simulink model "Simulatore_COMAU_UBHAND_IV_manipolazione_interna"  (Simulink 7.6) (continued)
== system Simulatore_COMAU_UBHAND_IV_manipolazione_interna/Work Space Control/Grasp Planner/box/presa dal basso == (continued)
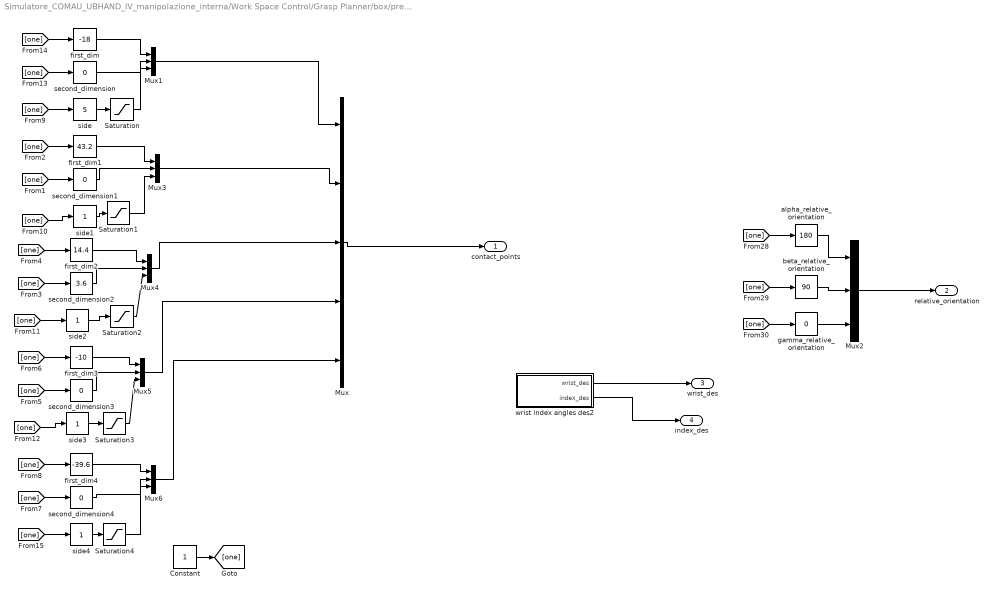
== system Simulatore_COMAU_UBHAND_IV_manipolazione_interna/Work Space Control/Grasp Planner/box/presa dall'alto ==
  Constant : Constant
  Mux : Mux  Inputs=5
  Mux{1..6} (6 alike) : Mux  Inputs=3
  Saturation{,1..4} (5 alike) : Saturate  UpperLimit=6  LowerLimit=1
  alpha_relative_orientation : simulink/Math Operations/Slider Gain  low=-180  gain=180  high=180
  beta_relative_orientation : simulink/Math Operations/Slider Gain  low=-180  gain=90  high=180
  first_dim : simulink/Math Operations/Slider Gain  low=-180  gain=-18  high=180
  first_dim1 : simulink/Math Operations/Slider Gain  low=-180  gain=43.2  high=180
  first_dim2 : simulink/Math Operations/Slider Gain  low=-180  gain=14.4  high=180
  first_dim3 : simulink/Math Operations/Slider Gain  low=-100  gain=-10  high=100
  first_dim4 : simulink/Math Operations/Slider Gain  low=-180  gain=-39.6  high=180
  gamma_relative_orientation : simulink/Math Operations/Slider Gain  low=-180  gain=0  high=180
  second_dimension{,1,3..4} (4 alike) : simulink/Math Operations/Slider Gain  low=-180  gain=0  high=180
  second_dimension2 : simulink/Math Operations/Slider Gain  low=-180  gain=3.6  high=180
  side : simulink/Math Operations/Slider Gain  low=-94  gain=5  high=6
  side{1..4} (4 alike) : simulink/Math Operations/Slider Gain  low=-94  gain=1  high=6
  wrist index angles des2 : Subsystem
  contact_points : Outport
  relative_orientation : Outport  Port=2
  wrist_des : Outport  Port=3
  index_des : Outport  Port=4
  wires:
  [one] -> alpha_relative_orientation/1
  [one] -> beta_relative_orientation/1
  [one] -> gamma_relative_orientation/1
  alpha_relative_orientation/1 -> Mux2/1
  beta_relative_orientation/1 -> Mux2/2
  gamma_relative_orientation/1 -> Mux2/3
  Mux2/1 -> relative_orientation/1
  Constant/1 -> [one]
  wrist index angles des2/1(wrist_des) -> wrist_des/1
  wrist index angles des2/2(index_des) -> index_des/1
  Mux/1 -> contact_points/1
  [one] -> second_dimension/1
  [one] -> first_dim/1
  first_dim/1 -> Mux1/1
  second_dimension/1 -> Mux1/2
  Mux1/1 -> Mux/1
  side/1 -> Saturation/1
  [one] -> second_dimension1/1
  [one] -> first_dim1/1
  first_dim1/1 -> Mux3/1
  second_dimension1/1 -> Mux3/2
  side1/1 -> Saturation1/1
  [one] -> second_dimension2/1
  [one] -> first_dim2/1
  first_dim2/1 -> Mux4/1
  second_dimension2/1 -> Mux4/2
  side2/1 -> Saturation2/1
  [one] -> second_dimension3/1
  [one] -> first_dim3/1
  first_dim3/1 -> Mux5/1
  second_dimension3/1 -> Mux5/2
  side3/1 -> Saturation3/1
  [one] -> second_dimension4/1
  [one] -> first_dim4/1
  first_dim4/1 -> Mux6/1
  second_dimension4/1 -> Mux6/2
  side4/1 -> Saturation4/1
  Mux3/1 -> Mux/2
  Mux4/1 -> Mux/3
  Mux5/1 -> Mux/4
  Mux6/1 -> Mux/5
  [one] -> side/1
  [one] -> side1/1
  [one] -> side2/1
  [one] -> side3/1
  [one] -> side4/1
  Saturation/1 -> Mux1/3
  Saturation1/1 -> Mux3/3
  Saturation2/1 -> Mux4/3
  Saturation3/1 -> Mux5/3
  Saturation4/1 -> Mux6/3

== system Simulatore_COMAU_UBHAND_IV_manipolazione_interna/Work Space Control/Grasp Planner/box/punti di contatto box ==
  parallelipedo_size : Inport
  sfera_pos : Inport  Port=2
  object_hand_relative_orientation : Inport  Port=3
  object_motion_parameters : Inport  Port=4
  teta_phi : Inport  Port=5
  Constant : Constant
  Demux : Demux  Outputs=5
  object rotation matrix : Subsystem
  punti di contatto sfera : Subsystem
  contact_tips_xyz : Outport
  wires:
  parallelipedo_size/1 -> punti di contatto sfera/6(box_size)
  punti di contatto sfera/1(contact_tips_xyz) -> contact_tips_xyz/1
  Constant/1 -> [one]
  object rotation matrix/1(T_object_center) -> punti di contatto sfera/7(T_object)
  sfera_pos/1 -> object rotation matrix/1(box_pos)
  object_hand_relative_orientation/1 -> object rotation matrix/2(object_hand_relative_orientation)
  object_motion_parameters/1 -> object rotation matrix/3(object_motion_parameters)
  teta_phi/1 -> Demux/1
  Demux/1 -> punti di contatto sfera/1(teta/z thumb)
  Demux/2 -> punti di contatto sfera/2(teta/z index)
  Demux/3 -> punti di contatto sfera/3(teta/z middle)
  Demux/4 -> punti di contatto sfera/4(teta/z ring)
  Demux/5 -> punti di contatto sfera/5(teta/z little)

== system Simulatore_COMAU_UBHAND_IV_manipolazione_interna/Work Space Control/Grasp Planner/cilindro/Subsystem ==
  obj_rotat_manip : Inport
  obj_trasl_manip : Inport  Port=2
  Constant1 : Constant  Value=[0 0 0 1]  VectorParams1D=off
  Matrix Concatenate : Concatenate  Mode=Multidimensional array  ConcatenateDimension=2
  Matrix Concatenate1 : Concatenate  Mode=Multidimensional array
  R_end_effector : Subsystem
  obj_manip : Outport
  tag scope: R_cilindro_manip=global
  wires:
  Constant1/1 -> Matrix Concatenate1/2
  Matrix Concatenate/1 -> Matrix Concatenate1/1
  R_end_effector/1(R_end_effector) -> Matrix Concatenate/1, [R_cilindro_manip]
  obj_rotat_manip/1 -> R_end_effector/1(alpha,beta,gamma)
  obj_trasl_manip/1 -> Matrix Concatenate/2
  Matrix Concatenate1/1 -> obj_manip/1

== system Simulatore_COMAU_UBHAND_IV_manipolazione_interna/Work Space Control/Grasp Planner/cilindro/presa da destra ==
  Add{,1..3,6..8} (7 alike) : Sum
  Constant : Constant
  Mux : Mux  Inputs=10
  Mux2 : Mux  Inputs=3
  alpha_relative_orientation : simulink/Math Operations/Slider Gain  low=-180  gain=90  high=180
  beta_relative_orientation : simulink/Math Operations/Slider Gain  low=-180  gain=90  high=180
  gamma_relative_orientation : simulink/Math Operations/Slider Gain  low=-180  gain=90  high=180
  teta : simulink/Math Operations/Slider Gain  low=-180  gain=115.2  high=180
  teta1 : simulink/Math Operations/Slider Gain  low=-180  gain=32.4  high=180
  teta2 : simulink/Math Operations/Slider Gain  low=-180  gain=0  high=180
  teta3 : simulink/Math Operations/Slider Gain  low=-180  gain=0  high=180
  teta4 : simulink/Math Operations/Slider Gain  low=-180  gain=0  high=180
  wrist index angles des2 : Subsystem
  z : simulink/Math Operations/Slider Gain  low=-100  gain=60  high=500
  z1 : simulink/Math Operations/Slider Gain  low=-100  gain=-4e-006  high=500
  z2 : simulink/Math Operations/Slider Gain  low=-100  gain=-24  high=500
  z3 : simulink/Math Operations/Slider Gain  low=-100  gain=-48  high=500
  z4 : simulink/Math Operations/Slider Gain  low=-100  gain=-72  high=500
  contact_points : Outport
  relative_orientation : Outport  Port=2
  wrist_des : Outport  Port=3
  index_des : Outport  Port=4
  wires:
  Mux2/1 -> relative_orientation/1
  gamma_relative_orientation/1 -> Mux2/3
  beta_relative_orientation/1 -> Mux2/2
  alpha_relative_orientation/1 -> Mux2/1
  [one] -> gamma_relative_orientation/1
  [one] -> beta_relative_orientation/1
  [one] -> alpha_relative_orientation/1
  Mux/1 -> contact_points/1
  [one] -> teta4/1
  [one] -> z4/1
  [one] -> teta3/1
  [one] -> z3/1
  [one] -> teta2/1
  [one] -> z2/1
  [one] -> teta1/1
  [one] -> z1/1
  [one] -> teta/1
  [one] -> z/1
  Constant/1 -> [one]
  wrist index angles des2/1(wrist_des) -> wrist_des/1
  wrist index angles des2/2(index_des) -> index_des/1
  z4/1 -> Add3/2
  Add3/1 -> Mux/10
  z3/1 -> Add2/2
  Add2/1 -> Mux/8
  z2/1 -> Add1/2
  Add1/1 -> Mux/6
  z1/1 -> Mux/4, Add/2, Add1/1, Add2/1, Add3/1
  teta1/1 -> Mux/3, Add6/1, Add7/1, Add8/1
  teta4/1 -> Add8/2
  Add8/1 -> Mux/9
  Add7/1 -> Mux/7
  teta3/1 -> Add7/2
  teta2/1 -> Add6/2
  Add6/1 -> Mux/5
  teta/1 -> Mux/1
  z/1 -> Add/1
  Add/1 -> Mux/2

== system Simulatore_COMAU_UBHAND_IV_manipolazione_interna/Work Space Control/Grasp Planner/cilindro/presa dal basso ==
  Add{,1..4,6..8} (8 alike) : Sum
  Constant : Constant
  Mux : Mux  Inputs=10
  Mux2 : Mux  Inputs=3
  alpha_relative_orientation : simulink/Math Operations/Slider Gain  low=-180  gain=180  high=180
  beta_relative_orientation : simulink/Math Operations/Slider Gain  low=-180  gain=90  high=180
  gamma_relative_orientation : simulink/Math Operations/Slider Gain  low=-180  gain=90  high=180
  teta : simulink/Math Operations/Slider Gain  low=-180  gain=-46.8  high=180
  teta1 : simulink/Math Operations/Slider Gain  low=-180  gain=-46.8  high=180
  teta2 : simulink/Math Operations/Slider Gain  low=-180  gain=39.6  high=180
  teta3 : simulink/Math Operations/Slider Gain  low=-180  gain=79.2  high=180
  teta4 : simulink/Math Operations/Slider Gain  low=-180  gain=126  high=180
  wrist index angles des2 : Subsystem
  z : simulink/Math Operations/Slider Gain  low=-100  gain=-42  high=500
  z1 : simulink/Math Operations/Slider Gain  low=-100  gain=-18  high=500
  z2 : simulink/Math Operations/Slider Gain  low=-100  gain=-6  high=500
  z3 : simulink/Math Operations/Slider Gain  low=-100  gain=-6  high=500
  z4 : simulink/Math Operations/Slider Gain  low=-100  gain=-12  high=500
  contact_points : Outport
  relative_orientation : Outport  Port=2
  wrist_des : Outport  Port=3
  index_des : Outport  Port=4
  wires:
  [one] -> z/1
  [one] -> teta/1
  [one] -> z1/1
  [one] -> teta1/1
  [one] -> z2/1
  [one] -> teta2/1
  [one] -> z3/1
  [one] -> teta3/1
  [one] -> z4/1
  [one] -> teta4/1
  [one] -> alpha_relative_orientation/1
  [one] -> beta_relative_orientation/1
  [one] -> gamma_relative_orientation/1
  alpha_relative_orientation/1 -> Mux2/1
  beta_relative_orientation/1 -> Mux2/2
  gamma_relative_orientation/1 -> Mux2/3
  Mux2/1 -> relative_orientation/1
  Constant/1 -> [one]
  wrist index angles des2/1(wrist_des) -> wrist_des/1
  wrist index angles des2/2(index_des) -> index_des/1
  Mux/1 -> contact_points/1
  z4/1 -> Add3/2
  Add3/1 -> Mux/10
  z3/1 -> Add2/2
  Add2/1 -> Mux/8
  z2/1 -> Add1/2
  Add1/1 -> Mux/6
  z1/1 -> Mux/4, Add/2, Add1/1, Add2/1, Add3/1
  teta1/1 -> Mux/3, Add6/1, Add7/1, Add8/1, Add4/2
  teta4/1 -> Add8/2
  Add8/1 -> Mux/9
  Add7/1 -> Mux/7
  teta3/1 -> Add7/2
  teta2/1 -> Add6/2
  Add6/1 -> Mux/5
  z/1 -> Add/1
  Add/1 -> Mux/2
  teta/1 -> Add4/1
  Add4/1 -> Mux/1

== system Simulatore_COMAU_UBHAND_IV_manipolazione_interna/Work Space Control/Grasp Planner/cilindro/presa dall'alto ==
  Add{,1..4,6..8} (8 alike) : Sum
  Constant : Constant
  Mux : Mux  Inputs=10
  Mux2 : Mux  Inputs=3
  alpha_relative_orientation : simulink/Math Operations/Slider Gain  low=-180  gain=180  high=180
  beta_relative_orientation : simulink/Math Operations/Slider Gain  low=-180  gain=90  high=180
  gamma_relative_orientation : simulink/Math Operations/Slider Gain  low=-180  gain=0  high=180
  index_angles_des : Display
  teta : simulink/Math Operations/Slider Gain  low=-180  gain=86.4  high=180
  teta1 : simulink/Math Operations/Slider Gain  low=-180  gain=54  high=180
  teta2 : simulink/Math Operations/Slider Gain  low=-180  gain=-36  high=180
  teta3 : simulink/Math Operations/Slider Gain  low=-180  gain=-79.2  high=180
  teta4 : simulink/Math Operations/Slider Gain  low=-180  gain=-147.6  high=180
  wrist index angles des2 : Subsystem
  wrist_angles_des : Display
  z : simulink/Math Operations/Slider Gain  low=-100  gain=-4  high=100
  z1 : simulink/Math Operations/Slider Gain  low=-100  gain=180  high=500
  z2 : simulink/Math Operations/Slider Gain  low=-100  gain=0  high=100
  z3 : simulink/Math Operations/Slider Gain  low=-100  gain=0  high=500
  z4 : simulink/Math Operations/Slider Gain  low=-200  gain=0  high=500
  contact_points : Outport
  relative_orientation : Outport  Port=2
  wrist_des : Outport  Port=3
  index_des : Outport  Port=4
  wires:
  [one] -> z/1
  [one] -> teta/1
  [one] -> z1/1
  [one] -> teta1/1
  [one] -> z2/1
  [one] -> teta2/1
  [one] -> z3/1
  [one] -> teta3/1
  [one] -> z4/1
  [one] -> teta4/1
  [one] -> alpha_relative_orientation/1
  [one] -> beta_relative_orientation/1
  [one] -> gamma_relative_orientation/1
  alpha_relative_orientation/1 -> Mux2/1
  beta_relative_orientation/1 -> Mux2/2
  gamma_relative_orientation/1 -> Mux2/3
  Mux2/1 -> relative_orientation/1
  Constant/1 -> [one]
  wrist index angles des2/1(wrist_des) -> wrist_des/1, wrist_angles_des/1
  wrist index angles des2/2(index_des) -> index_des/1, index_angles_des/1
  Mux/1 -> contact_points/1
  z4/1 -> Add3/2
  Add3/1 -> Mux/10
  z3/1 -> Add2/2
  Add2/1 -> Mux/8
  z2/1 -> Add1/2
  Add1/1 -> Mux/6
  z1/1 -> Mux/4, Add/2, Add1/1, Add2/1, Add3/1
  teta1/1 -> Mux/3, Add6/1, Add7/1, Add8/1, Add4/2
  teta4/1 -> Add8/2
  Add8/1 -> Mux/9
  Add7/1 -> Mux/7
  teta3/1 -> Add7/2
  teta2/1 -> Add6/2
  Add6/1 -> Mux/5
  z/1 -> Add/1
  Add/1 -> Mux/2
  teta/1 -> Add4/1
  Add4/1 -> Mux/1

== system Simulatore_COMAU_UBHAND_IV_manipolazione_interna/Work Space Control/Grasp Planner/cilindro/punti di contatto cilindro ==
  cilindro_size : Inport
  cilindro_pos : Inport  Port=2
  teta_z : Inport  Port=3
  object_hand_relative_orientation : Inport  Port=4
  object_motion_parameters : Inport  Port=5
  offset : Inport  Port=6
  object_manipulation : Inport  Port=7
  Angle Conversion{,1..4} (5 alike) : aerolibconvert2/Angle Conversion  IU=deg  OU=rad
  Constant : Constant
  Demux : Demux  Outputs=10
  Mux{,1..4} (5 alike) : Mux  Inputs=2
  object rotation matrix : Subsystem
  punti di contatto cilindro : Subsystem
  contact_tips_xyz : Outport
  index_xyz_0 : Outport  Port=2
  wires:
  cilindro_size/1 -> punti di contatto cilindro/6(cilindro_size)
  Mux/1 -> punti di contatto cilindro/1(teta/z thumb)
  Mux1/1 -> punti di contatto cilindro/2(teta/z index)
  Mux2/1 -> punti di contatto cilindro/3(teta/z middle)
  Mux3/1 -> punti di contatto cilindro/4(teta/z ring)
  Mux4/1 -> punti di contatto cilindro/5(teta/z little)
  punti di contatto cilindro/1(contact_tips_xyz) -> contact_tips_xyz/1
  Constant/1 -> [one]
  Angle Conversion/1 -> Mux/1
  Angle Conversion1/1 -> Mux1/1
  Angle Conversion2/1 -> Mux2/1
  Angle Conversion3/1 -> Mux3/1
  Angle Conversion4/1 -> Mux4/1
  object rotation matrix/1(T_object_center) -> punti di contatto cilindro/7(T_object)
  teta_z/1 -> Demux/1
  object_hand_relative_orientation/1 -> object rotation matrix/2(object_hand_relative_orientation)
  object_motion_parameters/1 -> object rotation matrix/3(object_motion_parameters)
  Demux/1 -> Angle Conversion/1
  Demux/2 -> Mux/2
  Demux/3 -> Angle Conversion1/1
  Demux/4 -> Mux1/2
  Demux/5 -> Angle Conversion2/1
  Demux/6 -> Mux2/2
  Demux/7 -> Angle Conversion3/1
  Demux/8 -> Mux3/2
  Demux/9 -> Angle Conversion4/1
  Demux/10 -> Mux4/2
  offset/1 -> punti di contatto cilindro/8(offset)
  cilindro_pos/1 -> object rotation matrix/1(cilindro_pos)
  object_manipulation/1 -> punti di contatto cilindro/9(object_manipulation)
  punti di contatto cilindro/2(index_xyz_0) -> index_xyz_0/1

== system Simulatore_COMAU_UBHAND_IV_manipolazione_interna/Work Space Control/Grasp Planner/sfera/presa da destra ==
  Constant : Constant
  Mux2 : Mux  Inputs=3
  alpha_relative_orientation : simulink/Math Operations/Slider Gain  low=-180  gain=180  high=180
  beta_relative_orientation : simulink/Math Operations/Slider Gain  low=-180  gain=90  high=180
  gamma_relative_orientation : simulink/Math Operations/Slider Gain  low=-180  gain=90  high=180
  relative_orientation : Outport
  wires:
  Constant/1 -> [one]
  [one] -> alpha_relative_orientation/1
  [one] -> beta_relative_orientation/1
  [one] -> gamma_relative_orientation/1
  alpha_relative_orientation/1 -> Mux2/1
  beta_relative_orientation/1 -> Mux2/2
  gamma_relative_orientation/1 -> Mux2/3
  Mux2/1 -> relative_orientation/1

== system Simulatore_COMAU_UBHAND_IV_manipolazione_interna/Work Space Control/Grasp Planner/sfera/presa dal basso ==
  Constant : Constant
  Mux2 : Mux  Inputs=3
  alpha_relative_orientation : simulink/Math Operations/Slider Gain  low=-180  gain=-90  high=180
  beta_relative_orientation : simulink/Math Operations/Slider Gain  low=-180  gain=90  high=180
  gamma_relative_orientation : simulink/Math Operations/Slider Gain  low=-180  gain=90  high=180
  relative_orientation : Outport
  wires:
  Constant/1 -> [one]
  Mux2/1 -> relative_orientation/1
  gamma_relative_orientation/1 -> Mux2/3
  beta_relative_orientation/1 -> Mux2/2
  alpha_relative_orientation/1 -> Mux2/1
  [one] -> gamma_relative_orientation/1
  [one] -> beta_relative_orientation/1
  [one] -> alpha_relative_orientation/1

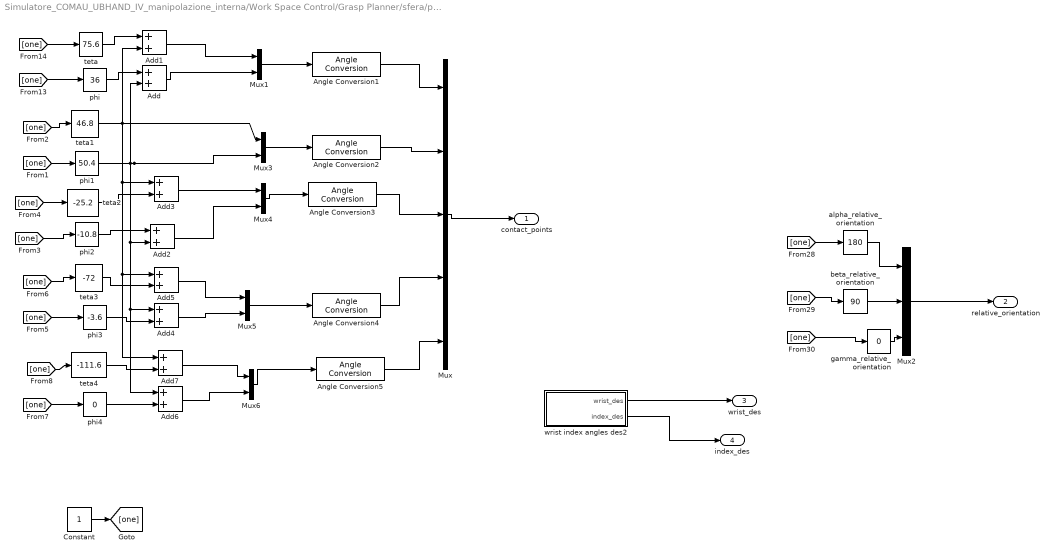
== system Simulatore_COMAU_UBHAND_IV_manipolazione_interna/Work Space Control/Grasp Planner/sfera/presa dall'alto ==
  Add{,1..7} (8 alike) : Sum
  Angle Conversion{1..5} (5 alike) : aerolibconvert2/Angle Conversion  IU=deg  OU=rad
  Constant : Constant
  Mux : Mux  Inputs=5
  Mux{1,3..6} (5 alike) : Mux  Inputs=2
  Mux2 : Mux  Inputs=3
  alpha_relative_orientation : simulink/Math Operations/Slider Gain  low=-180  gain=180  high=180
  beta_relative_orientation : simulink/Math Operations/Slider Gain  low=-180  gain=90  high=180
  gamma_relative_orientation : simulink/Math Operations/Slider Gain  low=-180  gain=0  high=180
  phi : simulink/Math Operations/Slider Gain  low=-180  gain=36  high=180
  phi1 : simulink/Math Operations/Slider Gain  low=-180  gain=50.4  high=180
  phi2 : simulink/Math Operations/Slider Gain  low=-180  gain=-10.8  high=180
  phi3 : simulink/Math Operations/Slider Gain  low=-180  gain=-3.6  high=180
  phi4 : simulink/Math Operations/Slider Gain  low=-180  gain=0  high=180
  teta : simulink/Math Operations/Slider Gain  low=-180  gain=75.6  high=180
  teta1 : simulink/Math Operations/Slider Gain  low=-180  gain=46.8  high=180
  teta2 : simulink/Math Operations/Slider Gain  low=-180  gain=-25.2  high=180
  teta3 : simulink/Math Operations/Slider Gain  low=-180  gain=-72  high=180
  teta4 : simulink/Math Operations/Slider Gain  low=-180  gain=-111.6  high=180
  wrist index angles des2 : Subsystem
  contact_points : Outport
  relative_orientation : Outport  Port=2
  wrist_des : Outport  Port=3
  index_des : Outport  Port=4
  wires:
  Mux/1 -> contact_points/1
  wrist index angles des2/2(index_des) -> index_des/1
  wrist index angles des2/1(wrist_des) -> wrist_des/1
  Constant/1 -> [one]
  Mux2/1 -> relative_orientation/1
  gamma_relative_orientation/1 -> Mux2/3
  beta_relative_orientation/1 -> Mux2/2
  alpha_relative_orientation/1 -> Mux2/1
  [one] -> gamma_relative_orientation/1
  [one] -> beta_relative_orientation/1
  [one] -> alpha_relative_orientation/1
  [one] -> phi/1
  [one] -> teta/1
  Mux1/1 -> Angle Conversion1/1
  [one] -> phi1/1
  [one] -> teta1/1
  teta1/1 -> Mux3/1, Add1/2, Add3/1, Add5/1, Add7/1
  [one] -> phi2/1
  [one] -> teta2/1
  [one] -> phi3/1
  [one] -> teta3/1
  [one] -> phi4/1
  [one] -> teta4/1
  Mux3/1 -> Angle Conversion2/1
  Mux4/1 -> Angle Conversion3/1
  Mux5/1 -> Angle Conversion4/1
  Mux6/1 -> Angle Conversion5/1
  phi1/1 -> Mux3/2, Add/2, Add6/1, Add4/1, Add2/2
  Angle Conversion1/1 -> Mux/1
  Angle Conversion2/1 -> Mux/2
  Angle Conversion3/1 -> Mux/3
  Angle Conversion4/1 -> Mux/4
  Angle Conversion5/1 -> Mux/5
  Add/1 -> Mux1/2
  Add1/1 -> Mux1/1
  teta4/1 -> Add7/2
  phi4/1 -> Add6/2
  Add6/1 -> Mux6/2
  Add7/1 -> Mux6/1
  Add4/1 -> Mux5/2
  Add5/1 -> Mux5/1
  Add2/1 -> Mux4/2
  Add3/1 -> Mux4/1
  phi/1 -> Add/1
  teta/1 -> Add1/1
  teta2/1 -> Add3/2
  phi3/1 -> Add4/2
  teta3/1 -> Add5/2
  phi2/1 -> Add2/1

== system Simulatore_COMAU_UBHAND_IV_manipolazione_interna/Work Space Control/Grasp Planner/sfera/punti di contatto sfera ==
  sfera_size : Inport
  sfera_pos : Inport  Port=2
  object_hand_relative_orientation : Inport  Port=3
  object_motion_parameters : Inport  Port=4
  teta_phi : Inport  Port=5
  Constant : Constant
  Demux : Demux  Outputs=5
  object rotation matrix : Subsystem
  punti di contatto sfera : Subsystem
  contact_tips_xyz : Outport
  wires:
  sfera_size/1 -> punti di contatto sfera/6(sfera_size)
  Demux/1 -> punti di contatto sfera/1(teta/z thumb)
  Demux/2 -> punti di contatto sfera/2(teta/z index)
  Demux/3 -> punti di contatto sfera/3(teta/z middle)
  Demux/5 -> punti di contatto sfera/5(teta/z little)
  Constant/1 -> [one]
  punti di contatto sfera/1(contact_tips_xyz) -> contact_tips_xyz/1
  object_motion_parameters/1 -> object rotation matrix/3(object_motion_parameters)
  object_hand_relative_orientation/1 -> object rotation matrix/2(object_hand_relative_orientation)
  sfera_pos/1 -> object rotation matrix/1(sfera_pos)
  object rotation matrix/1(T_object_center) -> punti di contatto sfera/7(T_object)
  teta_phi/1 -> Demux/1
  Demux/4 -> punti di contatto sfera/4(teta/z ring)

== system Simulatore_COMAU_UBHAND_IV_manipolazione_interna/Work Space Control/Inverse_kinematic/Inverse_kinematic_comau/R_0index ==
  wrist_angles_des1 : Inport
  index_angles_des1 : Inport  Port=2
  T_0_index : Inport  Port=3
  T_0_object : Inport  Port=4
  Matrix Multiply{1..4} (4 alike) : Product  Multiplication=Matrix(*)
  Multiport Switch : MultiPortSwitch  Inputs=2  zeroidx=off
  Multiport Switch1 : MultiPortSwitch  Inputs=2  zeroidx=off
  Multiport Switch2 : MultiPortSwitch  Inputs=2  zeroidx=off
  T6H : Constant  Value=T6HAND  VectorParams1D=off
  T6H1 : Constant  Value=T6HAND  VectorParams1D=off
  T_0_object1 : InportShadow  Port=4
  T_0index : Subsystem
  T_6_index_^-1 : Subsystem
  T_6_object^-1 : Subsystem
  T_index_object : Subsystem
  T_06 : Outport
  T_0H : Outport  Port=2
  tag scope: T_0EE=global
  wires:
  index_angles_des1/1 -> T_6_index_^-1/2(index_angles_des), T_0index/2(index_angles_des), T_6_object^-1/2(index_angles_des)
  Matrix Multiply1/1 -> Matrix Multiply2/1, Multiport Switch/2
  T6H/1 -> Matrix Multiply2/2
  wrist_angles_des1/1 -> T_0index/1(wrist_angles_des), T_6_index_^-1/1(wrist_angles_des), T_6_object^-1/1(wrist_angles_des)
  T_0_index/1 -> T_0index/3(T_0_end_effector), Multiport Switch2/2
  T_0index/1(T_0index) -> Matrix Multiply1/1, T_index_object/1(T_0_index)
  T_6_index_^-1/1(T_6_index^-1) -> Matrix Multiply1/2
  T_0_object/1 -> T_index_object/2(T_0_object), Multiport Switch2/3
  T_index_object/1(T_index_object) -> T_6_object^-1/3(T_index_object)
  T_0_object1/1 -> Matrix Multiply3/1
  T_6_object^-1/1(T_6_object^-1) -> Matrix Multiply3/2
  Multiport Switch/1 -> T_06/1
  Matrix Multiply3/1 -> Multiport Switch/3, Matrix Multiply4/1
  T6H1/1 -> Matrix Multiply4/2
  Matrix Multiply4/1 -> Multiport Switch1/3
  Matrix Multiply2/1 -> Multiport Switch1/2
  Multiport Switch1/1 -> T_0H/1
  [reference_mode] -> Multiport Switch/1, Multiport Switch1/1
  Multiport Switch2/1 -> [T_0EE]
  [reference_mode] -> Multiport Switch2/1

== system Simulatore_COMAU_UBHAND_IV_manipolazione_interna/Work Space Control/Inverse_kinematic/Inverse_kinematic_comau/Subsystem ==
  R36 : Inport
  In1 : Inport  Port=2
  Multiport Switch : MultiPortSwitch  Inputs=2  zeroidx=off
  q5 appartiene a [-180 0] : Subsystem
  q5 appartiene a [0 180] : Subsystem
  q[4..6] : Outport
  wires:
  R36/1 -> q5 appartiene a [0 180]/1(R_36), q5 appartiene a [-180 0]/1(R_36)
  q5 appartiene a [0 180]/1(q_[4..6]) -> Multiport Switch/2
  q5 appartiene a [-180 0]/1(q_[4..6]) -> Multiport Switch/3
  Multiport Switch/1 -> q[4..6]/1
  In1/1 -> Multiport Switch/1

== system Simulatore_COMAU_UBHAND_IV_manipolazione_interna/Work Space Control/Inverse_kinematic/Inverse_kinematic_comau/Subsystem1 ==
  end_effector_xyz : Inport
  R_0_end_effector : Inport  Port=2
  Constant : Constant  Value=[0 0 0 1]  VectorParams1D=off
  Matrix Concatenate : Concatenate  Mode=Multidimensional array  ConcatenateDimension=2
  Matrix Concatenate1 : Concatenate  Mode=Multidimensional array
  T_0_end_effector : Outport
  wires:
  Constant/1 -> Matrix Concatenate1/2
  Matrix Concatenate/1 -> Matrix Concatenate1/1
  R_0_end_effector/1 -> Matrix Concatenate/1
  end_effector_xyz/1 -> Matrix Concatenate/2
  Matrix Concatenate1/1 -> T_0_end_effector/1

== system Simulatore_COMAU_UBHAND_IV_manipolazione_interna/Work Space Control/Inverse_kinematic/Inverse_kinematic_comau/T_0_3 ==
  q[1..3] : Inport
  Add : Sum
  Add1 : Sum
  Add2 : Sum  Inputs=-+
  Constant : Constant  Value=[alpha_c(1) alpha_c(2) alpha_c(3) ]
  Constant1 : Constant  Value=teta_c(2)
  Constant2 : Constant  Value=[a_c(1) a_c(2) a_c(3)]
  Constant3 : Constant  Value=teta_c(3)
  Constant4 : Constant  Value=teta_c(1)
  Demux : Demux  Outputs=3
  Demux1 : Demux  Outputs=3
  Demux2 : Demux  Outputs=3
  Display3 : Display
  Matrix Multiply : Product  Multiplication=Matrix(*)
  Matrix Multiply1 : Product  Multiplication=Matrix(*)
  Submatrix : dspmtrx3/Submatrix  RowSpan=Range of rows  RowStartMode=Index  RowStartIndex=1  RowEndMode=Index  RowEndIndex=3  ColSpan=Range of columns  ColStartMode=Index  ColStartIndex=1  ColEndMode=Index  ColEndIndex=3
  Subsystem : Subsystem
  Subsystem1 : Subsystem
  Subsystem2 : Subsystem
  T_0_3 : Outport
  wires:
  Constant/1 -> Demux1/1
  Subsystem/1(Out1) -> Matrix Multiply/1
  Subsystem1/1(Out1) -> Matrix Multiply/2
  Matrix Multiply/1 -> Matrix Multiply1/1
  Subsystem2/1(Out1) -> Matrix Multiply1/2
  Matrix Multiply1/1 -> Submatrix/1
  Constant2/1 -> Demux2/1
  Demux2/1 -> Subsystem/3(a)
  Demux2/2 -> Subsystem1/3(a)
  Demux2/3 -> Subsystem2/3(a)
  Demux1/1 -> Subsystem/2(alpha)
  Demux1/2 -> Subsystem1/2(alpha)
  Demux1/3 -> Subsystem2/2(alpha)
  q[1..3]/1 -> Display3/1, Demux/1
  Add/1 -> Subsystem1/1(q)
  Constant1/1 -> Add/2
  Demux/2 -> Add/1
  Constant3/1 -> Add1/2
  Demux/3 -> Add1/1
  Add1/1 -> Subsystem2/1(q)
  Demux/1 -> Add2/1
  Add2/1 -> Subsystem/1(q)
  Constant4/1 -> Add2/2
  Submatrix/1 -> T_0_3/1

== system Simulatore_COMAU_UBHAND_IV_manipolazione_interna/Work Space Control/Inverse_kinematic/Inverse_kinematic_comau/calcR06,P06 ==
  4x4 end effector reference : Inport
  T6U : Inport  Port=2
  Display : Display
  P0U : dspmtrx3/Submatrix  RowSpan=Range of rows  RowStartMode=Index  RowStartIndex=1  RowEndMode=Index  RowEndIndex=3  ColSpan=One column  ColStartMode=Index  ColStartIndex=4  ColEndMode=Offset from last  ColEndIndex=3
  P6U : dspmtrx3/Submatrix  RowSpan=Range of rows  RowStartMode=Index  RowStartIndex=1  RowEndMode=Index  RowEndIndex=3  ColSpan=One column  ColStartMode=Index  ColStartIndex=4  ColEndMode=Index  ColEndIndex=3
  R0U : dspmtrx3/Submatrix  RowSpan=Range of rows  RowStartMode=Index  RowStartIndex=1  RowEndMode=Index  RowEndIndex=3  ColSpan=Range of columns  ColStartMode=Index  ColStartIndex=1  ColEndMode=Index  ColEndIndex=3
  R6U : dspmtrx3/Submatrix  RowSpan=Range of rows  RowStartMode=Index  RowStartIndex=1  RowEndMode=Index  RowEndIndex=3  ColSpan=Range of columns  ColStartMode=Index  ColStartIndex=1  ColEndMode=Index  ColEndIndex=3
  R6U' : PermuteDimensions
  R_06 : Product  Multiplication=Matrix(*)
  R_1 : Product  Multiplication=Matrix(*)
  Subtract : Sum  Inputs=+-
  R06 : Outport
  P06 : Outport  Port=2
  wires:
  T6U/1 -> R6U/1, P6U/1
  R6U/1 -> R6U'/1
  4x4 end effector reference/1 -> R0U/1, P0U/1
  R0U/1 -> R_06/1
  R6U'/1 -> R_06/2
  R_06/1 -> R06/1, R_1/1
  P6U/1 -> R_1/2
  R_1/1 -> Subtract/2
  P0U/1 -> Subtract/1
  Subtract/1 -> P06/1, Display/1

== system Simulatore_COMAU_UBHAND_IV_manipolazione_interna/Work Space Control/Inverse_kinematic/Inverse_kinematic_comau/calcR06,P1 ==
  R06 : Inport
  R03 : Inport  Port=2
  R03' : PermuteDimensions
  R_36 : Product  Multiplication=Matrix(*)
  R36 : Outport
  wires:
  R_36/1 -> R36/1
  R03'/1 -> R_36/1
  R03/1 -> R03'/1
  R06/1 -> R_36/2

== system Simulatore_COMAU_UBHAND_IV_manipolazione_interna/Work Space Control/Inverse_kinematic/Inverse_kinematic_comau/first solution ==
  P06 : Inport
  Mux : Mux  Inputs=3
  k : Subsystem
  q1 : Subsystem
  q2 : Subsystem
  q[1..3] : Outport
  wires:
  P06/1 -> q1/1(P06), k/2(P06), q2/1(P06)
  q1/1(q1) -> Mux/1, k/1(q1), q2/2(q1)
  Mux/1 -> q[1..3]/1
  k/1(q3) -> Mux/3
  q2/1(q2) -> Mux/2
  k/2(e_quadro1) -> q2/3(e_quadro)
